simulink model "PrkgClimaEgyMgr"  (R2022b) (continued)
== system PrkgClimaEgyMgr == (continued)
  VehModMngtGlbSafe1_Ngcc_VehModMngtGlbSafe1/1 -> PrkgClimaEgyMgr_Runnable_sys/67(VehModMngtGlbSafe1_Ngcc_VehModMngtGlbSafe1_read)
  WarnForEgyAllwdLimdDly_WarnForEgyAllwdLimdDly/1 -> PrkgClimaEgyMgr_Runnable_sys/68(WarnForEgyAllwdLimdDly_WarnForEgyAllwdLimdDly_read)
  WarnForPreCdngFctNotAvl_WarnForPreCdngFctNotAvl/1 -> PrkgClimaEgyMgr_Runnable_sys/69(WarnForPreCdngFctNotAvl_WarnForPreCdngFctNotAvl_read)
  WindIceDetdFrnt_Ngcc_WindIceDetdFrnt/1 -> PrkgClimaEgyMgr_Runnable_sys/70(WindIceDetdFrnt_Ngcc_WindIceDetdFrnt_read)
  WindIceDetdRe_Ngcc_WindIceDetdRe/1 -> PrkgClimaEgyMgr_Runnable_sys/71(WindIceDetdRe_Ngcc_WindIceDetdRe_read)
  CmptmtTRearRaw_CmptmtTRearRaw/1 -> PrkgClimaEgyMgr_Runnable_sys/72(CmptmtTRearRaw_CmptmtTRearRaw_read)
  RemClimaEgyExsrcReqPlus_RemClimaEgyExsrcReqPlus/1 -> PrkgClimaEgyMgr_Runnable_sys/73(RemClimaEgyExsrcReqPlus_RemClimaEgyExsrcReqPlus_read)
  PrkgClimaEgyMgr_Runnable_sys/1(ClimaCtrlVFCPreClimaHV_ClimaCtrlVFCPreClimaHV_write) -> ClimaCtrlVFCPreClimaHV_ClimaCtrlVFCPreClimaHV/1
  PrkgClimaEgyMgr_Runnable_sys/2(ClimaCtrlVFCPrkgClimaLV_ClimaCtrlVFCPrkgClimaLV_write) -> ClimaCtrlVFCPrkgClimaLV_ClimaCtrlVFCPrkgClimaLV/1
  PrkgClimaEgyMgr_Runnable_sys/3(CooltHeatrComReq_CooltHeatrComReq_write) -> CooltHeatrComReq_CooltHeatrComReq/1
  PrkgClimaEgyMgr_Runnable_sys/4(DataNewInPreClngStc_DataNewInPreClngStc_write) -> DataNewInPreClngStc_DataNewInPreClngStc/1
  PrkgClimaEgyMgr_Runnable_sys/5(DataNewInPrkgClimaFailr_DataNewInPrkgClimaFailr_write) -> DataNewInPrkgClimaFailr_DataNewInPrkgClimaFailr/1
  PrkgClimaEgyMgr_Runnable_sys/6(DataNewInPrkgClimaFinshd_DataNewInPrkgClimaFinshd_write) -> DataNewInPrkgClimaFinshd_DataNewInPrkgClimaFinshd/1
  DefrstHeatgForPrkgClimaEnad_DefrstHeatgForPrkgClimaEnad_merge/1 -> DefrstHeatgForPrkgClimaEnad_DefrstHeatgForPrkgClimaEnad/1
  PrkgClimaEgyMgr_Runnable_sys/8(DlyReqForTmrForPrkgClima_DlyReqForTmrForPrkgClima_write) -> DlyReqForTmrForPrkgClima_DlyReqForTmrForPrkgClima/1
  PrkgClimaEgyMgr_Runnable_sys/9(EgyAllwdForPrkgClima_EgyAllwdForPrkgClima_write) -> EgyAllwdForPrkgClima_EgyAllwdForPrkgClima/1
  PrkgClimaEgyMgr_Runnable_sys/10(EgyDesForClima_Ngcc_EgyDesForClima_write) -> EgyDesForClima_Ngcc_EgyDesForClima/1
  PrkgClimaEgyMgr_Runnable_sys/11(EgyPrioForPrkgClima_EgyPrioForPrkgClima_write) -> EgyPrioForPrkgClima_EgyPrioForPrkgClima/1
  PrkgClimaEgyMgr_Runnable_sys/12(FacForRtForPrkgClima_FacForRtForPrkgClima_write) -> FacForRtForPrkgClima_FacForRtForPrkgClima/1
  PrkgClimaEgyMgr_Runnable_sys/13(HvEgyDesForClima_Ngcc_HvEgyDesForClima_write) -> HvEgyDesForClima_Ngcc_HvEgyDesForClima/1
  PrkgClimaEgyMgr_Runnable_sys/14(OffForTLoForPostCdng_OffForTLoForPostCdng_write) -> OffForTLoForPostCdng_OffForTLoForPostCdng/1
  PrkgClimaEgyMgr_Runnable_sys/15(PreCdngWithHvBattActv_PreCdngWithHvBattActv_write) -> PreCdngWithHvBattActv_PreCdngWithHvBattActv/1
  PreClngStc_PreClngStc_merge/1 -> PreClngStc_PreClngStc/1
  PrkgClimaFailr_PrkgClimaFailr_merge/1 -> PrkgClimaFailr_PrkgClimaFailr/1
  PrkgClimaFinshd_PrkgClimaFinshd_merge/1 -> PrkgClimaFinshd_PrkgClimaFinshd/1
  PrkgClimaEgyMgr_Runnable_sys/19(PwrChrgDetdForPrkgHeatrElec_PwrChrgDetdForPrkgHeatrElec_write) -> PwrChrgDetdForPrkgHeatrElec_PwrChrgDetdForPrkgHeatrElec/1
  PrkgClimaEgyMgr_Runnable_sys/20(RtSdlForPrkgClima_RtSdlForPrkgClima_write) -> RtSdlForPrkgClima_RtSdlForPrkgClima/1
  PrkgClimaEgyMgr_Runnable_sys/21(WarnForEgyAllwdLimd_WarnForEgyAllwdLimd_write) -> WarnForEgyAllwdLimd_WarnForEgyAllwdLimd/1
  PrkgClimaEgyMgr_Runnable_sys/22(WarnForPrkgClimaNotAllwd_WarnForPrkgClimaNotAllwd_write) -> WarnForPrkgClimaNotAllwd_WarnForPrkgClimaNotAllwd/1
  PrkgClimaEgyMgr_Runnable_sys/18(PrkgClimaFinshd_PrkgClimaFinshd_write) -> [PrkgClimaFinshd_PrkgClimaFinshd_merge_Tag]
  [PrkgClimaFinshd_PrkgClimaFinshd_merge_Tag] -> PrkgClimaFinshd_PrkgClimaFinshd_merge/2
  PrkgClimaEgyMgr_Init/4(PrkgClimaFinshd_PrkgClimaFinshd_write) -> [PrkgClimaFinshd_PrkgClimaFinshd_merge_Tag1]
  [PrkgClimaFinshd_PrkgClimaFinshd_merge_Tag1] -> PrkgClimaFinshd_PrkgClimaFinshd_merge/1
  PrkgClimaEgyMgr_Runnable_sys/17(PrkgClimaFailr_PrkgClimaFailr_write) -> [PrkgClimaFailr_PrkgClimaFailr_merge_Tag]
  [PrkgClimaFailr_PrkgClimaFailr_merge_Tag] -> PrkgClimaFailr_PrkgClimaFailr_merge/2
  PrkgClimaEgyMgr_Init/3(PrkgClimaFailr_PrkgClimaFailr_write) -> [PrkgClimaFailr_PrkgClimaFailr_merge_Tag1]
  [PrkgClimaFailr_PrkgClimaFailr_merge_Tag1] -> PrkgClimaFailr_PrkgClimaFailr_merge/1
  PrkgClimaEgyMgr_Runnable_sys/16(PreClngStc_PreClngStc_write) -> [PreClngStc_PreClngStc_merge_Tag]
  [PreClngStc_PreClngStc_merge_Tag] -> PreClngStc_PreClngStc_merge/2
  PrkgClimaEgyMgr_Init/2(PreClngStc_PreClngStc_write) -> [PreClngStc_PreClngStc_merge_Tag1]
  [PreClngStc_PreClngStc_merge_Tag1] -> PreClngStc_PreClngStc_merge/1
  PrkgClimaEgyMgr_Runnable_sys/7(DefrstHeatgForPrkgClimaEnad_DefrstHeatgForPrkgClimaEnad_write) -> [DefrstHeatgForPrkgClimaEnad_DefrstHeatgForPrkg_0030795f1e24c17b]
  [DefrstHeatgForPrkgClimaEnad_DefrstHeatgForPrkg_0030795f1e24c17b] -> DefrstHeatgForPrkgClimaEnad_DefrstHeatgForPrkgClimaEnad_merge/2
  PrkgClimaEgyMgr_Init/1(DefrstHeatgForPrkgClimaEnad_DefrstHeatgForPrkgClimaEnad_write) -> [DefrstHeatgForPrkgClimaEnad_DefrstHeatgForPrkg_9b5e1f9afcbaaa8c]
  [DefrstHeatgForPrkgClimaEnad_DefrstHeatgForPrkg_9b5e1f9afcbaaa8c] -> DefrstHeatgForPrkgClimaEnad_DefrstHeatgForPrkgClimaEnad_merge/1
  PrkgClimaEgyMgr_Runnable/1 -> PrkgClimaEgyMgr_Runnable_sys/Trigger

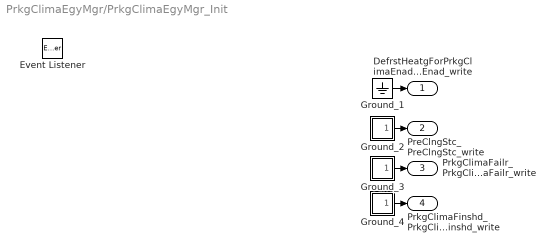
== system PrkgClimaEgyMgr/PrkgClimaEgyMgr_Init ==
  Event Listener : EventListener  EventName=reset
  Ground_1 : Ground
  Ground_2 : Subsystem
  Ground_3 : Subsystem
  Ground_4 : Subsystem
  DefrstHeatgForPrkgClimaEnad_DefrstHeatgForPrkgClimaEnad_write : Outport
  PreClngStc_PreClngStc_write : Outport  Port=2
  PrkgClimaFailr_PrkgClimaFailr_write : Outport  Port=3
  PrkgClimaFinshd_PrkgClimaFinshd_write : Outport  Port=4
  wires:
  Ground_1/1 -> DefrstHeatgForPrkgClimaEnad_DefrstHeatgForPrkgClimaEnad_write/1
  Ground_2/1(Out1) -> PreClngStc_PreClngStc_write/1
  Ground_3/1(Out1) -> PrkgClimaFailr_PrkgClimaFailr_write/1
  Ground_4/1(Out1) -> PrkgClimaFinshd_PrkgClimaFinshd_write/1

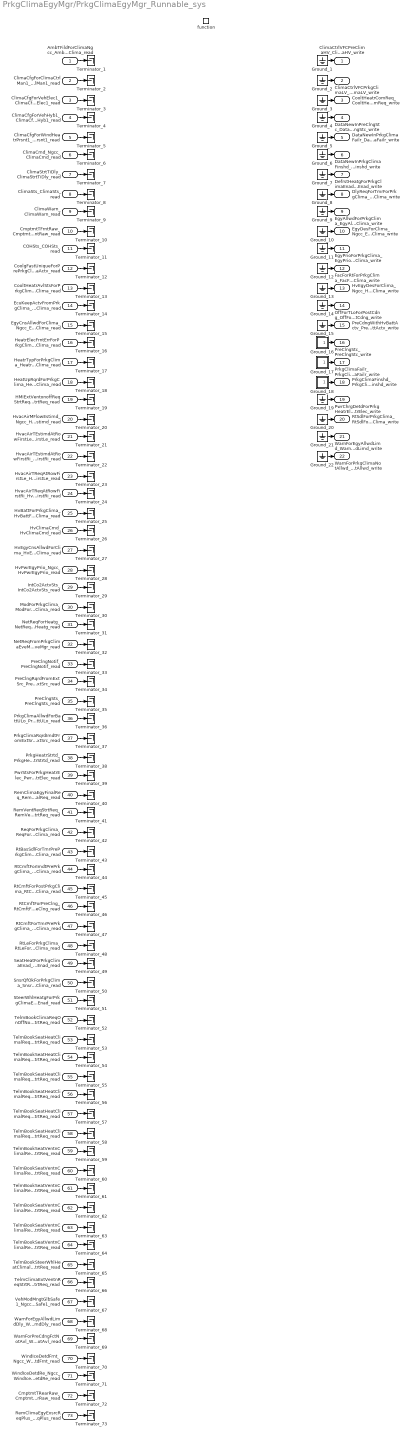
== system PrkgClimaEgyMgr/PrkgClimaEgyMgr_Runnable_sys ==
  AmbTFildForClimaNgcc_AmbTFildForClima_read : Inport
  ClimaCfgForClimaCtrlMan1_ClimaCfgForClimaCtrlMan1_read : Inport  Port=2
  ClimaCfgForVehElec1_ClimaCfgForVehElec1_read : Inport  Port=3
  ClimaCfgForVehHyb1_ClimaCfgForVehHyb1_read : Inport  Port=4
  ClimaCfgForWindHeatrPrsnt1_ClimaCfgForWindHeatrPrsnt1_read : Inport  Port=5
  ClimaCmd_Ngcc_ClimaCmd_read : Inport  Port=6
  ClimaStrtTiDly_ClimaStrtTiDly_read : Inport  Port=7
  ClimaSts_ClimaSts_read : Inport  Port=8
  ClimaWarn_ClimaWarn_read : Inport  Port=9
  CmptmtTFrntRaw_CmptmtTFrntRaw_read : Inport  Port=10
  COHSts_COHSts_read : Inport  Port=11
  CoolgFastUniqueForPrePrkgClimaActv_CoolgFastUniqueForPrePrkgClimaActv_read : Inport  Port=12
  CooltHeatrAvlStsForPrkgClima_CooltHeatrAvlStsForPrkgClima_read : Inport  Port=13
  EcuKeepActvFromPrkgClima_EcuKeepActvFromPrkgClima_read : Inport  Port=14
  EgyCnsAllwdForClima_Ngcc_EgyCnsAllwdForClima_read : Inport  Port=15
  HeatrElecFrntErrForPrkgClima_HeatrElecFrntErrForPrkgClima_read : Inport  Port=16
  HeatrTypForPrkgClima_HeatrTypForPrkgClima_read : Inport  Port=17
  HeatUpRqrdForPrkgClima_HeatUpRqrdForPrkgClima_read : Inport  Port=18
  HMIExtVentonoffReqStrtReq_HMIExtVentonoffReqStrtReq_read : Inport  Port=19
  HvacAirMFlowEstimd_Ngcc_HvacAirMFlowEstimd_read : Inport  Port=20
  HvacAirTEstimdAtRowFirstLe_HvacAirTEstimdAtRowFirstLe_read : Inport  Port=21
  HvacAirTEstimdAtRowFirstRi_HvacAirTEstimdAtRowFirstRi_read : Inport  Port=22
  HvacAirTReqAtRowFirstLe_HvacAirTReqAtRowFirstLe_read : Inport  Port=23
  HvacAirTReqAtRowFirstRi_HvacAirTReqAtRowFirstRi_read : Inport  Port=24
  HvBattForPrkgClima_HvBattForPrkgClima_read : Inport  Port=25
  HvClimaCmd_HvClimaCmd_read : Inport  Port=26
  HvEgyCnsAllwdForClima_HvEgyCnsAllwdForClima_read : Inport  Port=27
  HvPwrEgyPrio_Ngcc_HvPwrEgyPrio_read : Inport  Port=28
  IntCo2ActvSts_IntCo2ActvSts_read : Inport  Port=29
  ModForPrkgClima_ModForPrkgClima_read : Inport  Port=30
  NetReqForHeatg_NetReqForHeatg_read : Inport  Port=31
  NetReqFromPrkgClimaEveMgr_NetReqFromPrkgClimaEveMgr_read : Inport  Port=32
  PreClngNotif_PreClngNotif_read : Inport  Port=33
  PreClngRqrdFromExtSrc_PreClngRqrdFromExtSrc_read : Inport  Port=34
  PreClngSts_PreClngSts_read : Inport  Port=35
  PrkgClimaAllwdForBattULo_PrkgClimaAllwdForBattULo_read : Inport  Port=36
  PrkgClimaRqrdImdtFromExtSrc_PrkgClimaRqrdImdtFromExtSrc_read : Inport  Port=37
  PrkgHeatrStrtd_PrkgHeatrStrtd_read : Inport  Port=38
  PwrStsForPrkgHeatrElec_PwrStsForPrkgHeatrElec_read : Inport  Port=39
  RemClimaEgyFinalReq_RemClimaEgyFinalReq_read : Inport  Port=40
  RemVentReqStrtReq_RemVentReqStrtReq_read : Inport  Port=41
  ReqForPrkgClima_ReqForPrkgClima_read : Inport  Port=42
  RtBasSdlForTmrPrePrkgClima_RtBasSdlForTmrPrePrkgClima_read : Inport  Port=43
  RtCmftForImdtPrePrkgClima_RtCmftForImdtPrePrkgClima_read : Inport  Port=44
  RtCmftForPostPrkgClima_RtCmftForPostPrkgClima_read : Inport  Port=45
  RtCmftForPreClng_RtCmftForPreClng_read : Inport  Port=46
  RtCmftForTmrPrePrkgClima_RtCmftForTmrPrePrkgClima_read : Inport  Port=47
  RtLeForPrkgClima_RtLeForPrkgClima_read : Inport  Port=48
  SeatHeatForPrkgClimaEnad_SeatHeatForPrkgClimaEnad_read : Inport  Port=49
  SnsrQfOkForPrkgClima_SnsrQfOkForPrkgClima_read : Inport  Port=50
  SteerWhlHeatgForPrkgClimaEnad_SteerWhlHeatgForPrkgClimaEnad_read : Inport  Port=51
  TelmBookClimaReqOnOffNoReqStrtReq_TelmBookClimaReqOnOffNoReqStrtReq_read : Inport  Port=52
  TelmBookSeatHeatClimalReqDrvHeatClimaLvlStrtReq_TelmBookSeatHeatClimalReqDrvHeatClimaLvlStrtReq_read : Inport  Port=53
  TelmBookSeatHeatClimalReqPassHeatClimaLvlStrtReq_TelmBookSeatHeatClimalReqPassHeatClimaLvlStrtReq_read : Inport  Port=54
  TelmBookSeatHeatClimalReqSecLeHeatClimaLvlStrtReq_TelmBookSeatHeatClimalReqSecLeHeatClimaLvlStrtReq_read : Inport  Port=55
  TelmBookSeatHeatClimalReqSecRiHeatClimaLvlStrtReq_TelmBookSeatHeatClimalReqSecRiHeatClimaLvlStrtReq_read : Inport  Port=56
  TelmBookSeatHeatClimalReqThrdLeHeatClimaLvlStrtReq_TelmBookSeatHeatClimalReqThrdLeHeatClimaLvlStrtReq_read : Inport  Port=57
  TelmBookSeatHeatClimalReqThrdRiHeatClimaLvlStrtReq_TelmBookSeatHeatClimalReqThrdRiHeatClimaLvlStrtReq_read : Inport  Port=58
  TelmBookSeatVentnClimalReqDrvVentnClimaLvlStrtReq_TelmBookSeatVentnClimalReqDrvVentnClimaLvlStrtReq_read : Inport  Port=59
  TelmBookSeatVentnClimalReqPassVentnClimaLvlStrtReq_TelmBookSeatVentnClimalReqPassVentnClimaLvlStrtReq_read : Inport  Port=60
  TelmBookSeatVentnClimalReqSecLeVentnClimaLvlStrtReq_TelmBookSeatVentnClimalReqSecLeVentnClimaLvlStrtReq_read : Inport  Port=61
  TelmBookSeatVentnClimalReqSecRiVentnClimaLvlStrtReq_TelmBookSeatVentnClimalReqSecRiVentnClimaLvlStrtReq_read : Inport  Port=62
  TelmBookSeatVentnClimalReqThrdLeVentnClimaLvlStrtReq_TelmBookSeatVentnClimalReqThrdLeVentnClimaLvlStrtReq_read : Inport  Port=63
  TelmBookSeatVentnClimalReqThrdRiVentnClimaLvlStrtReq_TelmBookSeatVentnClimalReqThrdRiVentnClimaLvlStrtReq_read : Inport  Port=64
  TelmBookSteerWhlHeatClimalReqStrtReq_TelmBookSteerWhlHeatClimalReqStrtReq_read : Inport  Port=65
  TelmClimaExtVentnReqStrtReq_TelmClimaExtVentnReqStrtReq_read : Inport  Port=66
  VehModMngtGlbSafe1_Ngcc_VehModMngtGlbSafe1_read : Inport  Port=67
  WarnForEgyAllwdLimdDly_WarnForEgyAllwdLimdDly_read : Inport  Port=68
  WarnForPreCdngFctNotAvl_WarnForPreCdngFctNotAvl_read : Inport  Port=69
  WindIceDetdFrnt_Ngcc_WindIceDetdFrnt_read : Inport  Port=70
  WindIceDetdRe_Ngcc_WindIceDetdRe_read : Inport  Port=71
  CmptmtTRearRaw_CmptmtTRearRaw_read : Inport  Port=72
  RemClimaEgyExsrcReqPlus_RemClimaEgyExsrcReqPlus_read : Inport  Port=73
  function : TriggerPort  TriggerType=function-call  VariantControl=(inherit)  SampleTimeType=periodic  SampleTime=-1
  Ground_{1..15,19..22} (19 alike) : Ground
  Ground_16 : Subsystem
  Ground_17 : Subsystem
  Ground_18 : Subsystem
  Terminator_{1..73} (73 alike) : Terminator
  ClimaCtrlVFCPreClimaHV_ClimaCtrlVFCPreClimaHV_write : Outport
      settings: EnsureOutportIsVirtual=on
  ClimaCtrlVFCPrkgClimaLV_ClimaCtrlVFCPrkgClimaLV_write : Outport  Port=2
      settings: EnsureOutportIsVirtual=on
  CooltHeatrComReq_CooltHeatrComReq_write : Outport  Port=3
      settings: EnsureOutportIsVirtual=on
  DataNewInPreClngStc_DataNewInPreClngStc_write : Outport  Port=4
      settings: EnsureOutportIsVirtual=on
  DataNewInPrkgClimaFailr_DataNewInPrkgClimaFailr_write : Outport  Port=5
      settings: EnsureOutportIsVirtual=on
  DataNewInPrkgClimaFinshd_DataNewInPrkgClimaFinshd_write : Outport  Port=6
      settings: EnsureOutportIsVirtual=on
  DefrstHeatgForPrkgClimaEnad_DefrstHeatgForPrkgClimaEnad_write : Outport  Port=7
      settings: EnsureOutportIsVirtual=on
  DlyReqForTmrForPrkgClima_DlyReqForTmrForPrkgClima_write : Outport  Port=8
      settings: EnsureOutportIsVirtual=on
  EgyAllwdForPrkgClima_EgyAllwdForPrkgClima_write : Outport  Port=9
      settings: EnsureOutportIsVirtual=on
  EgyDesForClima_Ngcc_EgyDesForClima_write : Outport  Port=10
      settings: EnsureOutportIsVirtual=on
  EgyPrioForPrkgClima_EgyPrioForPrkgClima_write : Outport  Port=11
      settings: EnsureOutportIsVirtual=on
  FacForRtForPrkgClima_FacForRtForPrkgClima_write : Outport  Port=12
      settings: EnsureOutportIsVirtual=on
  HvEgyDesForClima_Ngcc_HvEgyDesForClima_write : Outport  Port=13
      settings: EnsureOutportIsVirtual=on
  OffForTLoForPostCdng_OffForTLoForPostCdng_write : Outport  Port=14
      settings: EnsureOutportIsVirtual=on
  PreCdngWithHvBattActv_PreCdngWithHvBattActv_write : Outport  Port=15
      settings: EnsureOutportIsVirtual=on
  PreClngStc_PreClngStc_write : Outport  Port=16
      settings: EnsureOutportIsVirtual=on
  PrkgClimaFailr_PrkgClimaFailr_write : Outport  Port=17
      settings: EnsureOutportIsVirtual=on
  PrkgClimaFinshd_PrkgClimaFinshd_write : Outport  Port=18
      settings: EnsureOutportIsVirtual=on
  PwrChrgDetdForPrkgHeatrElec_PwrChrgDetdForPrkgHeatrElec_write : Outport  Port=19
      settings: EnsureOutportIsVirtual=on
  RtSdlForPrkgClima_RtSdlForPrkgClima_write : Outport  Port=20
      settings: EnsureOutportIsVirtual=on
  WarnForEgyAllwdLimd_WarnForEgyAllwdLimd_write : Outport  Port=21
      settings: EnsureOutportIsVirtual=on
  WarnForPrkgClimaNotAllwd_WarnForPrkgClimaNotAllwd_write : Outport  Port=22
      settings: EnsureOutportIsVirtual=on
  wires:
  AmbTFildForClimaNgcc_AmbTFildForClima_read/1 -> Terminator_1/1
  ClimaCfgForClimaCtrlMan1_ClimaCfgForClimaCtrlMan1_read/1 -> Terminator_2/1
  ClimaCfgForVehElec1_ClimaCfgForVehElec1_read/1 -> Terminator_3/1
  ClimaCfgForVehHyb1_ClimaCfgForVehHyb1_read/1 -> Terminator_4/1
  ClimaCfgForWindHeatrPrsnt1_ClimaCfgForWindHeatrPrsnt1_read/1 -> Terminator_5/1
  ClimaCmd_Ngcc_ClimaCmd_read/1 -> Terminator_6/1
  ClimaStrtTiDly_ClimaStrtTiDly_read/1 -> Terminator_7/1
  ClimaSts_ClimaSts_read/1 -> Terminator_8/1
  ClimaWarn_ClimaWarn_read/1 -> Terminator_9/1
  CmptmtTFrntRaw_CmptmtTFrntRaw_read/1 -> Terminator_10/1
  COHSts_COHSts_read/1 -> Terminator_11/1
  CoolgFastUniqueForPrePrkgClimaActv_CoolgFastUniqueForPrePrkgClimaActv_read/1 -> Terminator_12/1
  CooltHeatrAvlStsForPrkgClima_CooltHeatrAvlStsForPrkgClima_read/1 -> Terminator_13/1
  EcuKeepActvFromPrkgClima_EcuKeepActvFromPrkgClima_read/1 -> Terminator_14/1
  EgyCnsAllwdForClima_Ngcc_EgyCnsAllwdForClima_read/1 -> Terminator_15/1
  HeatrElecFrntErrForPrkgClima_HeatrElecFrntErrForPrkgClima_read/1 -> Terminator_16/1
  HeatrTypForPrkgClima_HeatrTypForPrkgClima_read/1 -> Terminator_17/1
  HeatUpRqrdForPrkgClima_HeatUpRqrdForPrkgClima_read/1 -> Terminator_18/1
  HMIExtVentonoffReqStrtReq_HMIExtVentonoffReqStrtReq_read/1 -> Terminator_19/1
  HvacAirMFlowEstimd_Ngcc_HvacAirMFlowEstimd_read/1 -> Terminator_20/1
  HvacAirTEstimdAtRowFirstLe_HvacAirTEstimdAtRowFirstLe_read/1 -> Terminator_21/1
  HvacAirTEstimdAtRowFirstRi_HvacAirTEstimdAtRowFirstRi_read/1 -> Terminator_22/1
  HvacAirTReqAtRowFirstLe_HvacAirTReqAtRowFirstLe_read/1 -> Terminator_23/1
  HvacAirTReqAtRowFirstRi_HvacAirTReqAtRowFirstRi_read/1 -> Terminator_24/1
  HvBattForPrkgClima_HvBattForPrkgClima_read/1 -> Terminator_25/1
  HvClimaCmd_HvClimaCmd_read/1 -> Terminator_26/1
  HvEgyCnsAllwdForClima_HvEgyCnsAllwdForClima_read/1 -> Terminator_27/1
  HvPwrEgyPrio_Ngcc_HvPwrEgyPrio_read/1 -> Terminator_28/1
  IntCo2ActvSts_IntCo2ActvSts_read/1 -> Terminator_29/1
  ModForPrkgClima_ModForPrkgClima_read/1 -> Terminator_30/1
  NetReqForHeatg_NetReqForHeatg_read/1 -> Terminator_31/1
  NetReqFromPrkgClimaEveMgr_NetReqFromPrkgClimaEveMgr_read/1 -> Terminator_32/1
  PreClngNotif_PreClngNotif_read/1 -> Terminator_33/1
  PreClngRqrdFromExtSrc_PreClngRqrdFromExtSrc_read/1 -> Terminator_34/1
  PreClngSts_PreClngSts_read/1 -> Terminator_35/1
  PrkgClimaAllwdForBattULo_PrkgClimaAllwdForBattULo_read/1 -> Terminator_36/1
  PrkgClimaRqrdImdtFromExtSrc_PrkgClimaRqrdImdtFromExtSrc_read/1 -> Terminator_37/1
  PrkgHeatrStrtd_PrkgHeatrStrtd_read/1 -> Terminator_38/1
  PwrStsForPrkgHeatrElec_PwrStsForPrkgHeatrElec_read/1 -> Terminator_39/1
  RemClimaEgyFinalReq_RemClimaEgyFinalReq_read/1 -> Terminator_40/1
  RemVentReqStrtReq_RemVentReqStrtReq_read/1 -> Terminator_41/1
  ReqForPrkgClima_ReqForPrkgClima_read/1 -> Terminator_42/1
  RtBasSdlForTmrPrePrkgClima_RtBasSdlForTmrPrePrkgClima_read/1 -> Terminator_43/1
  RtCmftForImdtPrePrkgClima_RtCmftForImdtPrePrkgClima_read/1 -> Terminator_44/1
  RtCmftForPostPrkgClima_RtCmftForPostPrkgClima_read/1 -> Terminator_45/1
  RtCmftForPreClng_RtCmftForPreClng_read/1 -> Terminator_46/1
  RtCmftForTmrPrePrkgClima_RtCmftForTmrPrePrkgClima_read/1 -> Terminator_47/1
  RtLeForPrkgClima_RtLeForPrkgClima_read/1 -> Terminator_48/1
  SeatHeatForPrkgClimaEnad_SeatHeatForPrkgClimaEnad_read/1 -> Terminator_49/1
  SnsrQfOkForPrkgClima_SnsrQfOkForPrkgClima_read/1 -> Terminator_50/1
  SteerWhlHeatgForPrkgClimaEnad_SteerWhlHeatgForPrkgClimaEnad_read/1 -> Terminator_51/1
  TelmBookClimaReqOnOffNoReqStrtReq_TelmBookClimaReqOnOffNoReqStrtReq_read/1 -> Terminator_52/1
  TelmBookSeatHeatClimalReqDrvHeatClimaLvlStrtReq_TelmBookSeatHeatClimalReqDrvHeatClimaLvlStrtReq_read/1 -> Terminator_53/1
  TelmBookSeatHeatClimalReqPassHeatClimaLvlStrtReq_TelmBookSeatHeatClimalReqPassHeatClimaLvlStrtReq_read/1 -> Terminator_54/1
  TelmBookSeatHeatClimalReqSecLeHeatClimaLvlStrtReq_TelmBookSeatHeatClimalReqSecLeHeatClimaLvlStrtReq_read/1 -> Terminator_55/1
  TelmBookSeatHeatClimalReqSecRiHeatClimaLvlStrtReq_TelmBookSeatHeatClimalReqSecRiHeatClimaLvlStrtReq_read/1 -> Terminator_56/1
  TelmBookSeatHeatClimalReqThrdLeHeatClimaLvlStrtReq_TelmBookSeatHeatClimalReqThrdLeHeatClimaLvlStrtReq_read/1 -> Terminator_57/1
  TelmBookSeatHeatClimalReqThrdRiHeatClimaLvlStrtReq_TelmBookSeatHeatClimalReqThrdRiHeatClimaLvlStrtReq_read/1 -> Terminator_58/1
  TelmBookSeatVentnClimalReqDrvVentnClimaLvlStrtReq_TelmBookSeatVentnClimalReqDrvVentnClimaLvlStrtReq_read/1 -> Terminator_59/1
  TelmBookSeatVentnClimalReqPassVentnClimaLvlStrtReq_TelmBookSeatVentnClimalReqPassVentnClimaLvlStrtReq_read/1 -> Terminator_60/1
  TelmBookSeatVentnClimalReqSecLeVentnClimaLvlStrtReq_TelmBookSeatVentnClimalReqSecLeVentnClimaLvlStrtReq_read/1 -> Terminator_61/1
  TelmBookSeatVentnClimalReqSecRiVentnClimaLvlStrtReq_TelmBookSeatVentnClimalReqSecRiVentnClimaLvlStrtReq_read/1 -> Terminator_62/1
  TelmBookSeatVentnClimalReqThrdLeVentnClimaLvlStrtReq_TelmBookSeatVentnClimalReqThrdLeVentnClimaLvlStrtReq_read/1 -> Terminator_63/1
  TelmBookSeatVentnClimalReqThrdRiVentnClimaLvlStrtReq_TelmBookSeatVentnClimalReqThrdRiVentnClimaLvlStrtReq_read/1 -> Terminator_64/1
  TelmBookSteerWhlHeatClimalReqStrtReq_TelmBookSteerWhlHeatClimalReqStrtReq_read/1 -> Terminator_65/1
  TelmClimaExtVentnReqStrtReq_TelmClimaExtVentnReqStrtReq_read/1 -> Terminator_66/1
  VehModMngtGlbSafe1_Ngcc_VehModMngtGlbSafe1_read/1 -> Terminator_67/1
  WarnForEgyAllwdLimdDly_WarnForEgyAllwdLimdDly_read/1 -> Terminator_68/1
  WarnForPreCdngFctNotAvl_WarnForPreCdngFctNotAvl_read/1 -> Terminator_69/1
  WindIceDetdFrnt_Ngcc_WindIceDetdFrnt_read/1 -> Terminator_70/1
  WindIceDetdRe_Ngcc_WindIceDetdRe_read/1 -> Terminator_71/1
  CmptmtTRearRaw_CmptmtTRearRaw_read/1 -> Terminator_72/1
  RemClimaEgyExsrcReqPlus_RemClimaEgyExsrcReqPlus_read/1 -> Terminator_73/1
  Ground_1/1 -> ClimaCtrlVFCPreClimaHV_ClimaCtrlVFCPreClimaHV_write/1
  Ground_2/1 -> ClimaCtrlVFCPrkgClimaLV_ClimaCtrlVFCPrkgClimaLV_write/1
  Ground_3/1 -> CooltHeatrComReq_CooltHeatrComReq_write/1
  Ground_4/1 -> DataNewInPreClngStc_DataNewInPreClngStc_write/1
  Ground_5/1 -> DataNewInPrkgClimaFailr_DataNewInPrkgClimaFailr_write/1
  Ground_6/1 -> DataNewInPrkgClimaFinshd_DataNewInPrkgClimaFinshd_write/1
  Ground_7/1 -> DefrstHeatgForPrkgClimaEnad_DefrstHeatgForPrkgClimaEnad_write/1
  Ground_8/1 -> DlyReqForTmrForPrkgClima_DlyReqForTmrForPrkgClima_write/1
  Ground_9/1 -> EgyAllwdForPrkgClima_EgyAllwdForPrkgClima_write/1
  Ground_10/1 -> EgyDesForClima_Ngcc_EgyDesForClima_write/1
  Ground_11/1 -> EgyPrioForPrkgClima_EgyPrioForPrkgClima_write/1
  Ground_12/1 -> FacForRtForPrkgClima_FacForRtForPrkgClima_write/1
  Ground_13/1 -> HvEgyDesForClima_Ngcc_HvEgyDesForClima_write/1
  Ground_14/1 -> OffForTLoForPostCdng_OffForTLoForPostCdng_write/1
  Ground_15/1 -> PreCdngWithHvBattActv_PreCdngWithHvBattActv_write/1
  Ground_16/1(Out1) -> PreClngStc_PreClngStc_write/1
  Ground_17/1(Out1) -> PrkgClimaFailr_PrkgClimaFailr_write/1
  Ground_18/1(Out1) -> PrkgClimaFinshd_PrkgClimaFinshd_write/1
  Ground_19/1 -> PwrChrgDetdForPrkgHeatrElec_PwrChrgDetdForPrkgHeatrElec_write/1
  Ground_20/1 -> RtSdlForPrkgClima_RtSdlForPrkgClima_write/1
  Ground_21/1 -> WarnForEgyAllwdLimd_WarnForEgyAllwdLimd_write/1
  Ground_22/1 -> WarnForPrkgClimaNotAllwd_WarnForPrkgClimaNotAllwd_write/1

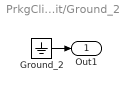
== system PrkgClimaEgyMgr/PrkgClimaEgyMgr_Init/Ground_2 ==
  Ground_2 : Ground
  Out1 : Outport  OutDataTypeStr=Bus: PreClngStcTyp  PortDimensions=1
  wires:
  Ground_2/1 -> Out1/1

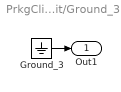
== system PrkgClimaEgyMgr/PrkgClimaEgyMgr_Init/Ground_3 ==
  Ground_3 : Ground
  Out1 : Outport  OutDataTypeStr=Bus: PrkgClimaFailrTyp  PortDimensions=1
  wires:
  Ground_3/1 -> Out1/1

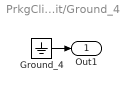
== system PrkgClimaEgyMgr/PrkgClimaEgyMgr_Init/Ground_4 ==
  Ground_4 : Ground
  Out1 : Outport  OutDataTypeStr=Bus: PrkgClimaFinshdTyp  PortDimensions=1
  wires:
  Ground_4/1 -> Out1/1

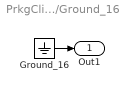
== system PrkgClimaEgyMgr/PrkgClimaEgyMgr_Runnable_sys/Ground_16 ==
  Ground_16 : Ground
  Out1 : Outport  OutDataTypeStr=Bus: PreClngStcTyp  PortDimensions=1
  wires:
  Ground_16/1 -> Out1/1

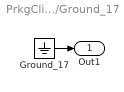
== system PrkgClimaEgyMgr/PrkgClimaEgyMgr_Runnable_sys/Ground_17 ==
  Ground_17 : Ground
  Out1 : Outport  OutDataTypeStr=Bus: PrkgClimaFailrTyp  PortDimensions=1
  wires:
  Ground_17/1 -> Out1/1

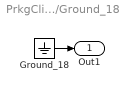
== system PrkgClimaEgyMgr/PrkgClimaEgyMgr_Runnable_sys/Ground_18 ==
  Ground_18 : Ground
  Out1 : Outport  OutDataTypeStr=Bus: PrkgClimaFinshdTyp  PortDimensions=1
  wires:
  Ground_18/1 -> Out1/1
